simulink model "MCVT_Integration"  (R2020a)
settings: Solver=ode8  StartTime=0.0  StopTime=T_end  FixedStep=dt  RelTol=1e-3  EnableMultiTasking=on
values from neighbouring files (symbols this model uses):
  assumed_power_voltage = 1.25e2    (= 125)    [MCVT_Input_File.m]    (also in MCVT_Run_QuickCheck.m)
  battery_power_toggle = 1    [MCVT_Input_File.m]    (also in MCVT_Run_QuickCheck.m)
  battery_trickle_charge_param = 0.1    [MCVT_Input_File.m]    (also in MCVT_Run_QuickCheck.m)
  battery_trickle_leakage_param = 0.01    [MCVT_Input_File.m]    (also in MCVT_Run_QuickCheck.m)
  damage_case_id = 3    [MCVT_Input_File.m]    (also in MCVT_Run_QuickCheck.m = 1)
  dt = 1/fs    (= 0.001)    [MCVT_Run_QuickCheck.m]    (also in MCVT_Sim_Set.m)
  dt_2 = 1/fs_2    (= 0.001)    [MCVT_Run_QuickCheck.m]    (also in MCVT_Sim_Set.m)
  dt_3 = 1/fs_3    (= 0.001)    [MCVT_Run_QuickCheck.m]    (also in MCVT_Sim_Set.m)
  dt_5 = 1/fs_5    (= 0.005)    [MCVT_Run_QuickCheck.m]    (also in MCVT_Sim_Set.m)
  dt_8 = 1/fs_8    (= 0.05)    [MCVT_Run_QuickCheck.m]    (also in MCVT_Sim_Set.m)
  dt_9 = 1/fs_9    (= 0.001)    [MCVT_Run_QuickCheck.m]    (also in MCVT_Sim_Set.m)
  dust_met_duration = 3    [MCVT_Input_File.m]    (also in MCVT_Run_QuickCheck.m = 2)
  ECLSS_indoor_set_temperature = 298.15    [MCVT_Input_File.m]    (also in MCVT_Run_QuickCheck.m)
  energy_store_max_o = 150    [MCVT_Input_File.m]    (also in MCVT_Run_QuickCheck.m)
  energy_store_o = 120    [MCVT_Input_File.m]    (also in MCVT_Run_QuickCheck.m)
  fs = 1000    [MCVT_Run_QuickCheck.m]    (also in MCVT_Sim_Set.m)
  gain_charge_param = 1    [MCVT_Input_File.m]    (also in MCVT_Run_QuickCheck.m)
  ground_temperature = 373    [MCVT_Habitat_Design_Input_File.m]    (also in MCVT_Run_QuickCheck.m)
  I_max_charge = 800    [MCVT_Input_File.m]    (also in MCVT_Run_QuickCheck.m)
  I_max_discharge = 800    [MCVT_Input_File.m]    (also in MCVT_Run_QuickCheck.m)
  initial_pressure = 1e5    (= 100000)    [MCVT_Input_File.m]    (also in MCVT_Run_QuickCheck.m)
  initial_temperature = 295    [MCVT_Input_File.m]    (also in MCVT_Run_QuickCheck.m)
  interior_leak_rate = 0.00415    [MCVT_Input_File.m]    (also in MCVT_Run_QuickCheck.m)
  launch_dust_accum_start_time = 10    [MCVT_Input_File.m]    (also in MCVT_Run_QuickCheck.m = 2)
  launch_dust_accum_stop_time = 12    [MCVT_Input_File.m]    (also in MCVT_Run_QuickCheck.m = 3)
  meteorite_impact_time = 2    [MCVT_Input_File.m]    (also in MCVT_Run_QuickCheck.m = 1)
  nuclear_power_toggle = 1    [MCVT_Input_File.m]    (also in MCVT_Run_QuickCheck.m)
  print_interval = 30    [MCVT_Input_File.m]
  SOC_lower_thres = 0.5    [MCVT_Input_File.m]    (also in MCVT_Run_QuickCheck.m)
  SOC_upper_thres = 0.9    [MCVT_Input_File.m]    (also in MCVT_Run_QuickCheck.m)
  solar_power_toggle = 1    [MCVT_Input_File.m]    (also in MCVT_Run_QuickCheck.m)
  space_temperature = 4    [MCVT_Habitat_Design_Input_File.m]    (also in MCVT_Run_QuickCheck.m)
  Start_angle = 90    [MCVT_Input_File.m]    (also in MCVT_Run_QuickCheck.m)
undefined symbols (not defined in the file or in its folder): A_flow_restrictor, ACTIVITY_LOOKUP_TABLE, air, Aref, Bmap, Bmap2, Bmap3, comms, DiscIntNeverNeededParam, DmgSPL, dust_parameters, ECLSS_dust_damage_repair_time, ECLSS_heater_capacity, ECLSS_IPS_restrictor, ECLSS_IPS_setpointPressure, ECLSS_paint_damage_repair_time, ECLSS_radiatorLoop_discreteNumber, ECLSS_Vst, emptyActivity, expected_heat_add, FI, habitat, HIEM2SMM, HIEM2STM, HIEMDamageElements, impact_numbering, initial_SPLT_damage_index, input_air, input_Cond, input_CP, input_discretization, input_EV, input_Evap, input_radiatorLoop, K_ECLSS_dust_single, K_ECLSS_paint_single, K_Structure_damage_single, Kref, kW_to_W, meteoroidDustDep, mFRF, ndelays, nominalDustDep, noverlap, nuclear_toggle_gain, output_limits, panel_paint0, Ps0, reference_temperature, RepairTime_ECLSS_factor, Sensor_Location_SMM, SMM_T0, SMM_T1, SMM_T2, SMM_T3, SMM_T4, SMM_T4_0, SMM_T4_1, SMM_T4_2, solar_FDD_K ... (+73)
libraries: neural x350, simulink x39, xpcutilitieslib x7, xpcrtudplib x7, dspstat3 x1
(part 4 of 43)

== system MCVT_Integration/Sys8 - Interior Environment/Habitat Interior Environment Model/Temperature Sensors ==
  note: Sensors_RTD_ECLSS_InputLoc
  note: Sensors_RTD_Zenith
  note: Sensors_RTD_Lef tB ottom
  note: Switch Control
      | 1 - Switch ON
      | 2 - Switch OFF
  note: Switch Control
      | 1 - Switch ON
      | 2 - Switch OFF
  note: Switch Control
      | 1 - Switch ON
      | 2 - Switch OFF
  Temperature (K) : Inport
  Power_Input (kW) : Inport  Port=2
  " Resistance Temperature Detector" : Subsystem
  " Resistance Temperature Detector1" : Subsystem
  " Resistance Temperature Detector2" : Subsystem
  " Switch ON/OFF Control Number" : MATLAB Function  TreatAsAtomicUnit=on
  " Switch ON/OFF Control Number1" : MATLAB Function  TreatAsAtomicUnit=on
  " Switch ON/OFF Control Number2" : MATLAB Function  TreatAsAtomicUnit=on
  Add : Sum  Inputs=+-
  Constant1 : Constant  Value=273.15
  Gain : Gain  Gain=1/3
  Memory3 : Memory  InitialCondition=initial_temperature-273.15  InheritSampleTime=on
  Memory4 : Memory  InitialCondition=initial_temperature-273.15  InheritSampleTime=on
  Memory5 : Memory  InitialCondition=initial_temperature-273.15  InheritSampleTime=on
  Mux : Mux  Inputs=2
  Mux1 : Mux  Inputs=2
  Mux2 : Mux  Inputs=2
  Mux3 : Mux  Inputs=3
  Scope : Scope
  Sensor Input to CDCM : MATLAB Function  TreatAsAtomicUnit=on
  Sensor Placement : MATLAB Function  TreatAsAtomicUnit=on
  Sum : Sum  Inputs=+++  AccumDataTypeStr=double
  Measured_Temperature (K) : Outport
  Power_Consumption (kW) : Outport  Port=2
  ECLSS_Measured_Temperature (K) : Outport  Port=3
  wires:
  " Resistance Temperature Detector2"/1(Physical Interdependency - OUT) -> Mux2/1  "Actual Temperature"
  " Resistance Temperature Detector"/2(Cyber Interdependency - OUT) -> Sensor Input to CDCM/1(ECLSS), ECLSS_Measured_Temperature (K)/1, Mux/2  "Measured Temperature"
  Memory3/1 -> " Resistance Temperature Detector"/1(Physical Interdependency - IN)
  " Switch ON/OFF Control Number1"/1(Switch) -> " Resistance Temperature Detector1"/2(Switch Control)
  Memory5/1 -> " Resistance Temperature Detector2"/1(Physical Interdependency - IN)
  " Resistance Temperature Detector1"/2(Cyber Interdependency - OUT) -> Sensor Input to CDCM/2(Zenith), Mux1/2  "Measured Temperature"
  " Resistance Temperature Detector"/1(Physical Interdependency - OUT) -> Mux/1  "Actual Temperature"
  " Resistance Temperature Detector2"/2(Cyber Interdependency - OUT) -> Sensor Input to CDCM/3(LeftBottom), Mux2/2  "Measured Temperature"
  Sensor Placement/3(LeftBottom) -> Memory5/1
  " Switch ON/OFF Control Number2"/1(Switch) -> " Resistance Temperature Detector2"/2(Switch Control)
  " Resistance Temperature Detector1"/1(Physical Interdependency - OUT) -> Mux1/1  "Actual Temperature"
  Memory4/1 -> " Resistance Temperature Detector1"/1(Physical Interdependency - IN)
  Sensor Placement/2(Zenith) -> Memory4/1
  Sensor Input to CDCM/1(Air_Temp_Sensed) -> Measured_Temperature (K)/1
  " Resistance Temperature Detector"/3(Power_Usage (kW)) -> Mux3/1, Sum/1
  " Resistance Temperature Detector1"/3(Power_Usage (kW)) -> Mux3/2, Sum/2
  " Resistance Temperature Detector2"/3(Power_Usage (kW)) -> Sum/3, Mux3/3
  Sum/1 -> Power_Consumption (kW)/1
  Gain/1 -> " Switch ON/OFF Control Number1"/1(Power), " Resistance Temperature Detector"/3(Power_Supply (kW)), " Switch ON/OFF Control Number"/1(Power), " Resistance Temperature Detector1"/3(Power_Supply (kW)), " Resistance Temperature Detector2"/3(Power_Supply (kW)), " Switch ON/OFF Control Number2"/1(Power)
  Temperature (K)/1 -> Add/1
  Add/1 -> Sensor Placement/1(Air_Temp)
  Constant1/1 -> Add/2
  " Switch ON/OFF Control Number"/1(Switch) -> " Resistance Temperature Detector"/2(Switch Control)
  Power_Input (kW)/1 -> Gain/1
  Sensor Placement/1(ECLSS) -> Scope/1, Memory3/1

== system MCVT_Integration/Sys9 - Exterior Environment/Exterior Environment/Dust ==
  exhaustEvent : Inport
  meteoroidEvent : Inport  Port=2
  nominalDustDep : Inport  Port=3
  meteoroidDustDep : Inport  Port=4
  d : Inport  Port=5
  input_L : Inport  Port=6
  DustDep : MATLAB Function  TreatAsAtomicUnit=on
  Unit Delay1 : UnitDelay  SampleTime=-1  HasFrameUpgradeWarning=on
  Unit Delay2 : UnitDelay  SampleTime=-1  HasFrameUpgradeWarning=on
  tao_total : Outport
  wires:
  d/1 -> DustDep/5(dist)
  input_L/1 -> DustDep/6(input_L)
  DustDep/1(tao_total) -> tao_total/1
  exhaustEvent/1 -> DustDep/1(exhaustEvent)
  nominalDustDep/1 -> DustDep/3(nominalDustDep)
  meteoroidEvent/1 -> DustDep/2(meteoroidEvent)
  meteoroidDustDep/1 -> DustDep/4(meteoroidDustDep)
  DustDep/2(count1) -> Unit Delay1/1
  Unit Delay1/1 -> DustDep/7(countp1)
  DustDep/3(count2) -> Unit Delay2/1
  Unit Delay2/1 -> DustDep/8(countp2)

== system MCVT_Integration/Sys9 - Exterior Environment/Exterior Environment/ExhaustEvent ==
  Add1 : Sum  Inputs=+-
  Step : Step  Time=launch_dust_accum_start_time  SampleTime=dt_9
  Step1 : Step  Time=launch_dust_accum_stop_time  SampleTime=dt_9
  Out1 : Outport
  wires:
  Add1/1 -> Out1/1
  Step/1 -> Add1/1
  Step1/1 -> Add1/2

== system MCVT_Integration/Sys9 - Exterior Environment/Exterior Environment/MeteoroidEvent ==
  Add1 : Sum  Inputs=+-
  Step : Step  Time=meteorite_impact_time  SampleTime=dt_9
  Step1 : Step  Time=meteorite_impact_time+dust_met_duration  SampleTime=dt_9
  meteoroidEvent : Outport
  wires:
  Add1/1 -> meteoroidEvent/1
  Step/1 -> Add1/1
  Step1/1 -> Add1/2

== system MCVT_Integration/Sys 6 - Health Management and Agents/ Agent System/Agent Module/Action Scheduler ==
  CURR_TODO : Inport
  Stateflow Module (re-factored) : Stateflow chart  TreatAsAtomicUnit=on
  Terminator : Terminator
  module1 : MATLAB Function  TreatAsAtomicUnit=on
  currAcvtDone : Outport
  currActID : Outport  Port=2
  currActParams : Outport  Port=3
  currActDone : Outport  Port=4
  actSampleTime : Outport  Port=5
  wires:
  CURR_TODO/1 -> module1/1(program)
  module1/2(todo_pgm) -> Stateflow Module (re-factored)/1(actionData)
  module1/3(nValidActions) -> Stateflow Module (re-factored)/2(numValidActions)
  Stateflow Module (re-factored)/1(actID) -> currActID/1
  Stateflow Module (re-factored)/2(actionParams) -> currActParams/1
  Stateflow Module (re-factored)/3(currAcvtDone) -> currAcvtDone/1
  Stateflow Module (re-factored)/4(currActDone) -> currActDone/1
  Stateflow Module (re-factored)/5(actSampleTime) -> actSampleTime/1
  module1/1(meta_pgm) -> Terminator/1

== system MCVT_Integration/Sys 6 - Health Management and Agents/ Agent System/Agent Module/Agent Memory ==
  currAcvtDone : Inport
  Intervention_Pgm : Inport  Port=2
  Linear Memory Buffer : MATLABSystem  System=LinearMemoryModule  maxMemorySlots=agent_memory_size  numMaxActions=numMaxActions  numMaxActionParams=numMaxActionParams  SimulateUsing=Code generation
      mask Type: LinearMemoryModule
      mask Display:
      | disp('LinearMemoryModule');
      | port_label('input',1,'C2Signal');
      | port_label('input',2,'currAcvtDone');
      | port_label('output',1,'currPgm');
      | port_label('output',2,'filledMemSlots');
      | port_label('output',3,'activityIDs');
      | port_label('output',4,'currAcvtID');
  currPgm : Outport
  filledMemSlots : Outport  Port=2
  activityIDs : Outport  Port=3
  currAcvtID : Outport  Port=4
  wires:
  Intervention_Pgm/1 -> Linear Memory Buffer/1
  Linear Memory Buffer/1 -> currPgm/1
  currAcvtDone/1 -> Linear Memory Buffer/2
  Linear Memory Buffer/2 -> filledMemSlots/1
  Linear Memory Buffer/3 -> activityIDs/1
  Linear Memory Buffer/4 -> currAcvtID/1

== system MCVT_Integration/Sys 6 - Health Management and Agents/ Agent System/Agent Module/Plant Model ==
  actionID : Inport
  actionParams : Inport  Port=2
  actionDone : Inport  Port=3
  actSampleTime : Inport  Port=4
  Agent System Object : MATLABSystem  System=AgentPlant  dt=dt_6  dischargeRate=R_batteryDischarge  mobDamageRate=R_mobDamage  lifeOfAgent=agent_life  SimulateUsing=Code generation
      mask Type: AgentPlant
      mask Display:
      | disp('AgentPlant');
      | port_label('input',1,'actionID');
      | port_label('input',2,'actionParams');
      | port_label('input',3,'actionDone');
      | port_label('input',4,'actSampleTime');
      | port_label('output',1,'States');
      | port_label('output',2,'CommsSubSys_Combined');
      | port_label('output',3,'doneFLAGS');
  States : Outport
  Comms_SubSys_Combined : Outport  Port=2
  action_done_flags : Outport  Port=3
  wires:
  actionID/1 -> Agent System Object/1  "actionID"
  actionDone/1 -> Agent System Object/3  "actionDone"
  actionParams/1 -> Agent System Object/2  "actionParams"
  actSampleTime/1 -> Agent System Object/4
  Agent System Object/1 -> States/1
  Agent System Object/2 -> Comms_SubSys_Combined/1
  Agent System Object/3 -> action_done_flags/1

== system MCVT_Integration/Sys 6 - Health Management and Agents/Decision Maker/Simulink Communication Modules/Agent//MCVT API from HMS ==
  MATLAB Function : MATLAB Function  TreatAsAtomicUnit=on
  Terminator5 : Terminator
  UDP_Receive_C2 : Subsystem
  HMS_Suggested_Activity : Outport
  wires:
  UDP_Receive_C2/2(Receive_output) -> MATLAB Function/1(u)
  UDP_Receive_C2/1(Receive_file) -> Terminator5/1
  UDP_Receive_C2/3(Receive_length) -> MATLAB Function/2(Receive_length)
  MATLAB Function/1(y) -> HMS_Suggested_Activity/1

== system MCVT_Integration/Sys 6 - Health Management and Agents/Decision Maker/Simulink Communication Modules/Agent//MCVT API to HMS ==
  note: Socket Information
  Agent_States : Inport
  Clock1 : Clock
  Constant1 : Constant  Value=comms.send.Agent.DBTableID
  Constant3 : Constant  Value=comms.send.Agent.PacketInfo
  Gain : Gain  Gain=fs
  Terminator : Terminator
  UDP_Send_C2 : Subsystem
  Vector Concatenate : Concatenate  NumInputs=3
  wires:
  Clock1/1 -> Gain/1
  Constant1/1 -> Vector Concatenate/1
  UDP_Send_C2/1(Send_file) -> Terminator/1
  Vector Concatenate/1 -> UDP_Send_C2/1(Input_Send)
  Gain/1 -> Vector Concatenate/2
  Constant3/1 -> UDP_Send_C2/2(Packet_Info)
  Agent_States/1 -> Vector Concatenate/3

== system MCVT_Integration/Sys 6 - Health Management and Agents/Decision Maker/Simulink Communication Modules/ECLSS_Dust//MCVT API to HMS ==
  note: Socket Definition
  note: FDD Information "Summarizer"
  ECLSS_Dust_xe : Inport
  Bus Creator : BusCreator  Inputs=3
  Bus Selector : BusSelector  OutputSignals=FDD_ECLSS_Dust_xe_avg
  Clock1 : Clock
  Constant : Constant  Value=comms.send.ECLSS.FDD_Dust.fsRatio
  Constant3 : Constant  Value=comms.send.ECLSS.FDD_Dust.PacketInfo
  Gain : Gain  Gain=fs
  Terminator : Terminator
  UDP_Send : Subsystem
  Vector Concatenate : Concatenate  NumInputs=3
  module2 : MATLAB Function  TreatAsAtomicUnit=on
  table_id : Constant  Value=comms.send.ECLSS.FDD_Dust.DBTableID
  wires:
  Constant/1 -> module2/2(buffSize)
  Gain/1 -> Vector Concatenate/2
  table_id/1 -> Vector Concatenate/1
  Vector Concatenate/1 -> UDP_Send/1(Input_Send)
  UDP_Send/1(Send_file) -> Terminator/1
  Clock1/1 -> Gain/1
  Constant3/1 -> UDP_Send/2(Packet_Info)
  ECLSS_Dust_xe/1 -> Bus Creator/1, module2/1(u_t)  "FDD_ECLSS_Dust_xe"
  module2/2(u_std) -> Bus Creator/3  "FDD_ECLSS_Dust_xe_std"
  module2/1(u_av) -> Bus Creator/2  "FDD_ECLSS_Dust_xe_avg"
  Bus Creator/1 -> [FDD_ECLSS_Dust_Summary]
  [FDD_ECLSS_Dust_Summary] -> Bus Selector/1
  Bus Selector/1 -> Vector Concatenate/3  "<FDD_ECLSS_Dust_xe_avg>"

== system MCVT_Integration/Sys 6 - Health Management and Agents/Decision Maker/Simulink Communication Modules/ECLSS_Paint//MCVT API to HMS ==
  note: Socket Information
  note: FDD Information "Summarizer"
  ECLSS_Paint_xe : Inport
  Bus Creator : BusCreator  Inputs=3
  Bus Selector : BusSelector  OutputSignals=FDD_ECLSS_Paint_xe_avg
  Clock1 : Clock
  Constant : Constant  Value=comms.send.ECLSS.FDD_Paint.fsRatio
  Constant3 : Constant  Value=comms.send.ECLSS.FDD_Paint.PacketInfo
  Gain : Gain  Gain=fs
  Terminator : Terminator
  UDP_Send : Subsystem
  Vector Concatenate : Concatenate  NumInputs=3
  module2 : MATLAB Function  TreatAsAtomicUnit=on
  table_id : Constant  Value=comms.send.ECLSS.FDD_Paint.DBTableID
  wires:
  Constant/1 -> module2/2(buffSize)
  ECLSS_Paint_xe/1 -> Bus Creator/1, module2/1(u_t)  "FDD_ECLSS_Paint_xe"
  Vector Concatenate/1 -> UDP_Send/1(Input_Send)
  Clock1/1 -> Gain/1
  Gain/1 -> Vector Concatenate/2
  UDP_Send/1(Send_file) -> Terminator/1
  Constant3/1 -> UDP_Send/2(Packet_Info)
  table_id/1 -> Vector Concatenate/1
  module2/1(u_av) -> Bus Creator/2  "FDD_ECLSS_Paint_xe_avg"
  module2/2(u_std) -> Bus Creator/3  "FDD_ECLSS_Paint_xe_std"
  Bus Creator/1 -> [FDD_ECLSS_Paint_Summary]
  [FDD_ECLSS_Paint_Summary] -> Bus Selector/1
  Bus Selector/1 -> Vector Concatenate/3  "<FDD_ECLSS_Paint_xe_avg>"

== system MCVT_Integration/Sys 6 - Health Management and Agents/Decision Maker/Simulink Communication Modules/NPG_Dust//MCVT API to HMS ==
  note: Socket Definition
  note: FDD Information "Summarizer"
  FDD_NPG_Dust : Inport
  Buffer Average : MATLAB Function  TreatAsAtomicUnit=on
  Bus Creator : BusCreator  Inputs=3
  Bus Selector : BusSelector  OutputSignals=FDD_NPG_Dust_avg
  Clock1 : Clock
  Constant : Constant  Value=comms.send.NPG.FDD_Dust.fsRatio
  Constant1 : Constant  Value=comms.send.NPG.FDD_Dust.DBTableID
  Constant3 : Constant  Value=comms.send.NPG.FDD_Dust.PacketInfo
  Gain : Gain  Gain=fs
  Gain1 : Gain  Gain=1/sub3_nuc_FDD_gain
  Terminator1 : Terminator
  UDP_Send : Subsystem
  Vector Concatenate : Concatenate  NumInputs=3
  wires:
  Constant/1 -> Buffer Average/2(buffSize)
  Constant1/1 -> Vector Concatenate/1
  Clock1/1 -> Gain/1
  Gain/1 -> Vector Concatenate/2
  UDP_Send/1(Send_file) -> Terminator1/1
  Constant3/1 -> UDP_Send/2(Packet_Info)
  Vector Concatenate/1 -> UDP_Send/1(Input_Send)
  Buffer Average/1(u_av) -> Bus Creator/2  "FDD_NPG_Dust_avg"
  Buffer Average/2(u_std) -> Bus Creator/3  "FDD_NPG_Dust_std"
  Bus Creator/1 -> [FDD_PG_Dust_Summary]
  [FDD_PG_Dust_Summary] -> Bus Selector/1
  Bus Selector/1 -> Vector Concatenate/3  "<FDD_NPG_Dust_avg>"
  Gain1/1 -> Bus Creator/1, Buffer Average/1(u_t)  "FDD_NPG_Dust"
  FDD_NPG_Dust/1 -> Gain1/1

== system MCVT_Integration/Sys 6 - Health Management and Agents/Decision Maker/Simulink Communication Modules/Solar_PV//MCVT API to HMS ==
  note: Socket Definition
  note: FDD Information "Summarizer"
  FDD_SPG_Dust_xe : Inport
  Buffer Average : MATLAB Function  TreatAsAtomicUnit=on
  Bus Creator : BusCreator  Inputs=3
  Bus Selector : BusSelector  OutputSignals=FDD_SPG_Dust_xe_avg
  Bus Selector1 : BusSelector  OutputSignals=FDD_SPG_Dust_xe,FDD_SPG_Dust_xe_avg,FDD_SPG_Dust_xe_std
  Clock1 : Clock
  Constant : Constant  Value=comms.send.SPG.FDD_Dust.fsRatio
  Constant1 : Constant  Value=comms.send.SPG.FDD_Dust.DBTableID
  Constant3 : Constant  Value=comms.send.SPG.FDD_Dust.PacketInfo
  Gain : Gain  Gain=fs
  Scope : Scope  NumInputPorts=3
  Terminator1 : Terminator
  UDP_Send : Subsystem
  Vector Concatenate : Concatenate  NumInputs=3
  wires:
  FDD_SPG_Dust_xe/1 -> Bus Creator/1, Buffer Average/1(u_t)  "FDD_SPG_Dust_xe"
  Constant/1 -> Buffer Average/2(buffSize)
  Constant1/1 -> Vector Concatenate/1
  Clock1/1 -> Gain/1
  Gain/1 -> Vector Concatenate/2
  UDP_Send/1(Send_file) -> Terminator1/1
  Constant3/1 -> UDP_Send/2(Packet_Info)
  Vector Concatenate/1 -> UDP_Send/1(Input_Send)
  Buffer Average/1(u_av) -> Bus Creator/2  "FDD_SPG_Dust_xe_avg"
  Buffer Average/2(u_std) -> Bus Creator/3  "FDD_SPG_Dust_xe_std"
  Bus Creator/1 -> [FDD_SPG_Dust_Summary]
  [FDD_SPG_Dust_Summary] -> Bus Selector/1
  Bus Selector/1 -> Vector Concatenate/3  "<FDD_SPG_Dust_xe_avg>"
  [FDD_SPG_Dust_Summary] -> Bus Selector1/1
  Bus Selector1/1 -> Scope/1  "<FDD_SPG_Dust_xe>"
  Bus Selector1/2 -> Scope/2  "<FDD_SPG_Dust_xe_avg>"
  Bus Selector1/3 -> Scope/3  "<FDD_SPG_Dust_xe_std>"

== system MCVT_Integration/Sys 6 - Health Management and Agents/Decision Maker/Simulink Communication Modules/Structure_Dmg//MCVT API to HMS ==
  note: Socket Definition
  note: FDD Information "Summarizer"
  Structure_FDD_Damage_xe : Inport
  Buffer Average : MATLAB Function  TreatAsAtomicUnit=on
  Bus Creator : BusCreator  Inputs=3
  Bus Selector : BusSelector  OutputSignals=Str_FDD_Dmg_xe,Str_FDD_Dmg_xe_avg,Str_FDD_Dmg_xe_std
  Bus Selector1 : BusSelector  OutputSignals=Str_FDD_Dmg_xe_avg
  Clock1 : Clock
  Constant : Constant  Value=comms.send.Str.FDD_Dmg.fsRatio
  Constant1 : Constant  Value=comms.send.Str.FDD_Dmg.DBTableID
  Constant3 : Constant  Value=comms.send.Str.FDD_Dmg.PacketInfo
  Gain : Gain  Gain=fs
  Structure Scope : Scope  NumInputPorts=3
  Terminator1 : Terminator
  UDP_Send : Subsystem
  Vector Concatenate : Concatenate  NumInputs=3
  wires:
  Structure_FDD_Damage_xe/1 -> Bus Creator/1, Buffer Average/1(u_t)  "Str_FDD_Dmg_xe"
  Constant/1 -> Buffer Average/2(buffSize)
  Buffer Average/2(u_std) -> Bus Creator/3  "Str_FDD_Dmg_xe_std"
  Constant1/1 -> Vector Concatenate/1
  Clock1/1 -> Gain/1
  Gain/1 -> Vector Concatenate/2
  UDP_Send/1(Send_file) -> Terminator1/1
  Constant3/1 -> UDP_Send/2(Packet_Info)
  Vector Concatenate/1 -> UDP_Send/1(Input_Send)
  Buffer Average/1(u_av) -> Bus Creator/2  "Str_FDD_Dmg_xe_avg"
  Bus Creator/1 -> [FDD_Str_Dmg_Summary]
  [FDD_Str_Dmg_Summary] -> Bus Selector/1
  Bus Selector/1 -> Structure Scope/1  "<Str_FDD_Dmg_xe>"
  Bus Selector/2 -> Structure Scope/2  "<Str_FDD_Dmg_xe_avg>"
  Bus Selector/3 -> Structure Scope/3  "<Str_FDD_Dmg_xe_std>"
  [FDD_Str_Dmg_Summary] -> Bus Selector1/1
  Bus Selector1/1 -> Vector Concatenate/3  "<Str_FDD_Dmg_xe_avg>"

== system MCVT_Integration/Sys2 - Structural System/Sys2_Structural_System/Fault Detection and Diagnosis (FDD) 2.3/Accelerometer ==
  Actural Acceleration : Inport
  Accelerometer Power Supply : Inport  Port=2
  Acceleration Delay : Subsystem
  Accelerometer : Subsystem
  Mux : Mux  Inputs=2
  Selector16 : Selector  InputPortWidth=9  IndexOptions=Index vector (dialog)  Indices=[6]  OutputSizes=1
  Selector17 : Selector  InputPortWidth=9  IndexOptions=Index vector (dialog)  Indices=[6]  OutputSizes=1
  Out2 : Outport
  wires:
  Acceleration Delay/1(Delayed Acceleration) -> Out2/1
  Selector17/1 -> Mux/2  "Measured Acceleration"
  Selector16/1 -> Mux/1  "Actural Acceleration"
  Actural Acceleration/1 -> Selector16/1, Accelerometer/1(Physical Interdependency - IN)
  Accelerometer/1(Cyber Interdependency - OUT) -> Acceleration Delay/1(Measured Acceleration), Selector17/1  "Measured Acceleration"
  Accelerometer Power Supply/1 -> Accelerometer/2(SensorPowerSupply)

== system MCVT_Integration/Sys2 - Structural System/Sys2_Structural_System/Fault Detection and Diagnosis (FDD) 2.3/Data Anomaly Detection ==
  "Measured Acceleration " : Inport
  "Trigger " : TriggerPort  VariantControl=Variant
  Data Anomaly Detection : MATLAB Function  TreatAsAtomicUnit=on
  indicator : Outport
  wires:
  "Measured Acceleration "/1 -> Data Anomaly Detection/1(Acc)
  Data Anomaly Detection/1(indicator) -> indicator/1

== system MCVT_Integration/Sys2 - Structural System/Sys2_Structural_System/Fault Detection and Diagnosis (FDD) 2.3/FRF from Accelerometer 1 ==
  Excitation (White Noise) : Inport
  Acceleration Response : Inport  Port=2
  "Trigger " : TriggerPort  VariantControl=Variant
  Constant : Constant  Value=w
  Constant1 : Constant  Value=mFRF
  Constant2 : Constant  Value=noverlap
  FRF Calculation : MATLAB Function  TreatAsAtomicUnit=on
  Out1 : Outport
  wires:
  Constant2/1 -> FRF Calculation/4(noverlap)
  Constant1/1 -> FRF Calculation/3(m)
  Constant/1 -> FRF Calculation/5(w)
  Acceleration Response/1 -> FRF Calculation/2(acc)
  Excitation (White Noise)/1 -> FRF Calculation/1(exc)
  FRF Calculation/1(frf) -> Out1/1

== system MCVT_Integration/Sys2 - Structural System/Sys2_Structural_System/Fault Detection and Diagnosis (FDD) 2.3/FRF from Accelerometer 2 ==
  Excitation (White Noise) : Inport
  Acceleration Response : Inport  Port=2
  "Trigger " : TriggerPort  VariantControl=Variant
  Constant : Constant  Value=w
  Constant1 : Constant  Value=mFRF
  Constant2 : Constant  Value=noverlap
  FRF Calculation : MATLAB Function  TreatAsAtomicUnit=on
  Out1 : Outport
  wires:
  Excitation (White Noise)/1 -> FRF Calculation/1(exc)
  Acceleration Response/1 -> FRF Calculation/2(acc)
  Constant/1 -> FRF Calculation/5(w)
  Constant1/1 -> FRF Calculation/3(m)
  Constant2/1 -> FRF Calculation/4(noverlap)
  FRF Calculation/1(frf) -> Out1/1

== system MCVT_Integration/Sys2 - Structural System/Sys2_Structural_System/Fault Detection and Diagnosis (FDD) 2.3/FRF from Accelerometer 3 ==
  Excitation (White Noise) : Inport
  Acceleration Response : Inport  Port=2
  "Trigger " : TriggerPort  VariantControl=Variant
  Constant : Constant  Value=w
  Constant1 : Constant  Value=mFRF
  Constant2 : Constant  Value=noverlap
  FRF Calculation : MATLAB Function  TreatAsAtomicUnit=on
  Out1 : Outport
  wires:
  Constant2/1 -> FRF Calculation/4(noverlap)
  Constant1/1 -> FRF Calculation/3(m)
  Constant/1 -> FRF Calculation/5(w)
  Acceleration Response/1 -> FRF Calculation/2(acc)
  Excitation (White Noise)/1 -> FRF Calculation/1(exc)
  FRF Calculation/1(frf) -> Out1/1

== system MCVT_Integration/Sys2 - Structural System/Sys2_Structural_System/Fault Detection and Diagnosis (FDD) 2.3/Pattern Recognition Neural Network ==
  FRF 1 : Inport
  FRF 2 : Inport  Port=2
  FRF 3 : Inport  Port=3
  "Trigger " : TriggerPort  VariantControl=Variant
  Matrix Concatenate : Concatenate  NumInputs=3  Mode=Multidimensional array  ConcatenateDimension=2
  Pattern Recognition Neural Network with 10% Noise : Subsystem
      mask help: Pattern Recognition Neural Network
      mask Display: e = 0.06;c1 = [120 150 230]/255;c2 = ([120 150 230]+0)/383;c3 = ([120 150 230]+128)/383;patch([0 1 1 0],[0 0 1 1],c1);patch([0 1 1 1-e 1-e 0+e],[0 0 1 1-e 0+e 0+e],c2);patch([0 0 1 1-e 0+e 0+e],[0 1 1 1-e 1-e 0+e],c3);plot([0 1 1 0 0],[0 0 1 1 0]);fprintf('NNET');port_label('input',1,' Input');port_label('output',1,'Output ');
  Damage Probabilities : Outport
  wires:
  Matrix Concatenate/1 -> Pattern Recognition Neural Network with 10% Noise/1(Input)
  FRF 1/1 -> Matrix Concatenate/1
  FRF 2/1 -> Matrix Concatenate/2
  FRF 3/1 -> Matrix Concatenate/3
  Pattern Recognition Neural Network with 10% Noise/1(Output) -> Damage Probabilities/1

== system MCVT_Integration/Sys2 - Structural System/Sys2_Structural_System/Fault Detection and Diagnosis (FDD) with Binary Classifer/Accelerometer ==
  Actural Acceleration : Inport
  Accelerometer Power Supply : Inport  Port=2
  Acceleration Delay : Subsystem
  Accelerometer : Subsystem
  Mux : Mux  Inputs=2
  Scope : Scope
  Selector16 : Selector  InputPortWidth=9  IndexOptions=Index vector (dialog)  Indices=[6]  OutputSizes=1
  Selector17 : Selector  InputPortWidth=9  IndexOptions=Index vector (dialog)  Indices=[6]  OutputSizes=1
  To Workspace : ToWorkspace  VariableName=Measured_Acc  SampleTime=-1
  Acceleration_delay : Outport
  Acceleration_origin : Outport  Port=2
  wires:
  Acceleration Delay/1(Delayed Acceleration) -> Acceleration_delay/1
  Selector17/1 -> Mux/2  "Measured Acceleration"
  Selector16/1 -> Mux/1  "Actural Acceleration"
  Actural Acceleration/1 -> Selector16/1, Accelerometer/1(Physical Interdependency - IN)
  Mux/1 -> Scope/1
  Accelerometer/1(Cyber Interdependency - OUT) -> Acceleration_origin/1, Acceleration Delay/1(Measured Acceleration), Selector17/1, To Workspace/1  "Measured Acceleration"
  Accelerometer Power Supply/1 -> Accelerometer/2(SensorPowerSupply)

== system MCVT_Integration/Sys2 - Structural System/Sys2_Structural_System/Fault Detection and Diagnosis (FDD) with Binary Classifer/Data Anomaly Detection ==
  "Measured Acceleration " : Inport
  "Trigger " : TriggerPort  VariantControl=Variant
  Data Anomaly Detection : MATLAB Function  TreatAsAtomicUnit=on
  To Workspace : ToWorkspace  Commented=on  VariableName=max_value  SampleTime=-1
  To Workspace1 : ToWorkspace  Commented=on  VariableName=num_non_zeros  SampleTime=-1
  To Workspace2 : ToWorkspace  Commented=on  VariableName=Data_Anomaly_Indicator  SampleTime=-1
  To Workspace3 : ToWorkspace  Commented=on  VariableName=y  SampleTime=-1
  indicator : Outport
  wires:
  "Measured Acceleration "/1 -> Data Anomaly Detection/1(Acc)
  Data Anomaly Detection/1(indicator) -> To Workspace2/1, indicator/1
  Data Anomaly Detection/2(max_value) -> To Workspace/1
  Data Anomaly Detection/3(num_nan) -> To Workspace1/1
  Data Anomaly Detection/4(y) -> To Workspace3/1

== system MCVT_Integration/Sys2 - Structural System/Sys2_Structural_System/Fault Detection and Diagnosis (FDD) with Binary Classifer/FRF from Accelerometer 1 ==
  Excitation (White Noise) : Inport
  Acceleration Response : Inport  Port=2
  "Trigger " : TriggerPort  VariantControl=Variant
  Constant : Constant  Value=w
  Constant1 : Constant  Value=mFRF
  Constant2 : Constant  Value=noverlap
  FRF Calculation : MATLAB Function  TreatAsAtomicUnit=on
  To Workspace1 : ToWorkspace  VariableName=FRF_Input_Exc_1  SampleTime=-1
  To Workspace2 : ToWorkspace  VariableName=FRF_Input_Acc_1  SampleTime=-1
  To Workspace3 : ToWorkspace  VariableName=FRF_1  SampleTime=-1
  Out1 : Outport
  wires:
  Constant2/1 -> FRF Calculation/4(noverlap)
  Constant1/1 -> FRF Calculation/3(m)
  Constant/1 -> FRF Calculation/5(w)
  Acceleration Response/1 -> FRF Calculation/2(acc), To Workspace2/1
  Excitation (White Noise)/1 -> FRF Calculation/1(exc), To Workspace1/1
  FRF Calculation/1(frf) -> To Workspace3/1, Out1/1

== system MCVT_Integration/Sys2 - Structural System/Sys2_Structural_System/Fault Detection and Diagnosis (FDD) with Binary Classifer/FRF from Accelerometer 2 ==
  Excitation (White Noise) : Inport
  Acceleration Response : Inport  Port=2
  "Trigger " : TriggerPort  VariantControl=Variant
  Constant : Constant  Value=w
  Constant1 : Constant  Value=mFRF
  Constant2 : Constant  Value=noverlap
  FRF Calculation : MATLAB Function  TreatAsAtomicUnit=on
  To Workspace1 : ToWorkspace  VariableName=FRF_Input_Exc_2  SampleTime=-1
  To Workspace2 : ToWorkspace  VariableName=FRF_Input_Acc_2  SampleTime=-1
  To Workspace3 : ToWorkspace  VariableName=FRF_2  SampleTime=-1
  Out1 : Outport
  wires:
  Excitation (White Noise)/1 -> To Workspace1/1, FRF Calculation/1(exc)
  Acceleration Response/1 -> To Workspace2/1, FRF Calculation/2(acc)
  Constant/1 -> FRF Calculation/5(w)
  Constant1/1 -> FRF Calculation/3(m)
  Constant2/1 -> FRF Calculation/4(noverlap)
  FRF Calculation/1(frf) -> To Workspace3/1, Out1/1

== system MCVT_Integration/Sys2 - Structural System/Sys2_Structural_System/Fault Detection and Diagnosis (FDD) with Binary Classifer/FRF from Accelerometer 3 ==
  Excitation (White Noise) : Inport
  Acceleration Response : Inport  Port=2
  "Trigger " : TriggerPort  VariantControl=Variant
  Constant : Constant  Value=w
  Constant1 : Constant  Value=mFRF
  Constant2 : Constant  Value=noverlap
  FRF Calculation : MATLAB Function  TreatAsAtomicUnit=on
  To Workspace1 : ToWorkspace  VariableName=FRF_Input_Exc_3  SampleTime=-1
  To Workspace2 : ToWorkspace  VariableName=FRF_Input_Acc_3  SampleTime=-1
  To Workspace3 : ToWorkspace  VariableName=FRF_3  SampleTime=-1
  Out1 : Outport
  wires:
  Constant2/1 -> FRF Calculation/4(noverlap)
  Constant1/1 -> FRF Calculation/3(m)
  Constant/1 -> FRF Calculation/5(w)
  Acceleration Response/1 -> FRF Calculation/2(acc), To Workspace2/1
  Excitation (White Noise)/1 -> FRF Calculation/1(exc), To Workspace1/1
  FRF Calculation/1(frf) -> To Workspace3/1, Out1/1

== system MCVT_Integration/Sys2 - Structural System/Sys2_Structural_System/Fault Detection and Diagnosis (FDD) with Binary Classifer/Pattern Recognition Neural Network ==
  FRF 1 : Inport
  FRF 2 : Inport  Port=2
  FRF 3 : Inport  Port=3
  "Trigger " : TriggerPort  VariantControl=Variant
  Matrix Concatenate : Concatenate  NumInputs=3  Mode=Multidimensional array  ConcatenateDimension=2
  Segment 1 : Subsystem
      mask help: Pattern Recognition Neural Network
      mask Display: e = 0.06;c1 = [120 150 230]/255;c2 = ([120 150 230]+0)/383;c3 = ([120 150 230]+128)/383;patch([0 1 1 0],[0 0 1 1],c1);patch([0 1 1 1-e 1-e 0+e],[0 0 1 1-e 0+e 0+e],c2);patch([0 0 1 1-e 0+e 0+e],[0 1 1 1-e 1-e 0+e],c3);plot([0 1 1 0 0],[0 0 1 1 0]);fprintf('NNET');port_label('input',1,' Input');port_label('output',1,'Output ');
  Segment 2 (2) : Subsystem
      mask help: Pattern Recognition Neural Network
      mask Display: e = 0.06;c1 = [120 150 230]/255;c2 = ([120 150 230]+0)/383;c3 = ([120 150 230]+128)/383;patch([0 1 1 0],[0 0 1 1],c1);patch([0 1 1 1-e 1-e 0+e],[0 0 1 1-e 0+e 0+e],c2);patch([0 0 1 1-e 0+e 0+e],[0 1 1 1-e 1-e 0+e],c3);plot([0 1 1 0 0],[0 0 1 1 0]);fprintf('NNET');port_label('input',1,' Input');port_label('output',1,'Output ');
  Segment 3 : Subsystem
      mask help: Pattern Recognition Neural Network
      mask Display: e = 0.06;c1 = [120 150 230]/255;c2 = ([120 150 230]+0)/383;c3 = ([120 150 230]+128)/383;patch([0 1 1 0],[0 0 1 1],c1);patch([0 1 1 1-e 1-e 0+e],[0 0 1 1-e 0+e 0+e],c2);patch([0 0 1 1-e 0+e 0+e],[0 1 1 1-e 1-e 0+e],c3);plot([0 1 1 0 0],[0 0 1 1 0]);fprintf('NNET');port_label('input',1,' Input');port_label('output',1,'Output ');
  Segment 4 : Subsystem
      mask help: Pattern Recognition Neural Network
      mask Display: e = 0.06;c1 = [120 150 230]/255;c2 = ([120 150 230]+0)/383;c3 = ([120 150 230]+128)/383;patch([0 1 1 0],[0 0 1 1],c1);patch([0 1 1 1-e 1-e 0+e],[0 0 1 1-e 0+e 0+e],c2);patch([0 0 1 1-e 0+e 0+e],[0 1 1 1-e 1-e 0+e],c3);plot([0 1 1 0 0],[0 0 1 1 0]);fprintf('NNET');port_label('input',1,' Input');port_label('output',1,'Output ');
  Segment 5 : Subsystem
      mask help: Pattern Recognition Neural Network
      mask Display: e = 0.06;c1 = [120 150 230]/255;c2 = ([120 150 230]+0)/383;c3 = ([120 150 230]+128)/383;patch([0 1 1 0],[0 0 1 1],c1);patch([0 1 1 1-e 1-e 0+e],[0 0 1 1-e 0+e 0+e],c2);patch([0 0 1 1-e 0+e 0+e],[0 1 1 1-e 1-e 0+e],c3);plot([0 1 1 0 0],[0 0 1 1 0]);fprintf('NNET');port_label('input',1,' Input');port_label('output',1,'Output ');
  To Workspace1 : ToWorkspace  VariableName=Damage_Probabilities_Segment_2_Noise_10  SampleTime=-1
  To Workspace2 : ToWorkspace  VariableName=Damage_Probabilities_Segment_3_Noise_10  SampleTime=-1
  To Workspace3 : ToWorkspace  VariableName=FRF  SampleTime=-1
  To Workspace4 : ToWorkspace  VariableName=Damage_Probabilities_Segment_4_Noise_10  SampleTime=-1
  To Workspace5 : ToWorkspace  VariableName=Damage_Probabilities_Segment_5_Noise_10  SampleTime=-1
  To Workspace7 : ToWorkspace  VariableName=Damage_Probabilities_Segment_1_Noise_10  SampleTime=-1
  Damage Probabilities Segment 1 : Outport
  Damage Probabilities Segment 2 : Outport  Port=2
  Damage Probabilities Segment 3 : Outport  Port=3
  Damage Probabilities Segment 4 : Outport  Port=4
  Damage Probabilities Segment 5 : Outport  Port=5
  wires:
  Matrix Concatenate/1 -> To Workspace3/1, Segment 3/1(Input), Segment 2 (2)/1(Input), Segment 1/1(Input), Segment 5/1(Input), Segment 4/1(Input)
  FRF 1/1 -> Matrix Concatenate/1
  FRF 2/1 -> Matrix Concatenate/2
  FRF 3/1 -> Matrix Concatenate/3
  Segment 1/1(Output) -> Damage Probabilities Segment 1/1, To Workspace7/1
  Segment 2 (2)/1(Output) -> Damage Probabilities Segment 2/1, To Workspace1/1
  Segment 3/1(Output) -> Damage Probabilities Segment 3/1, To Workspace2/1
  Segment 4/1(Output) -> Damage Probabilities Segment 4/1, To Workspace4/1
  Segment 5/1(Output) -> Damage Probabilities Segment 5/1, To Workspace5/1

== system MCVT_Integration/Sys2 - Structural System/Sys2_Structural_System/Fault Detection and Diagnosis (FDD) with Binary Classifer/Subsystem ==
  Acceleration : Inport
  Repair : Inport  Port=2
  Clock : Clock
  Constant : Constant  Value=dt_2
  Delay4 : Delay  DelayLength=500  InitialCondition=0
  Demux : Demux  Outputs=9
  Enabled Subsystem3 : Subsystem  TreatAsAtomicUnit=on
  Information Fusion1 : MATLAB Function  TreatAsAtomicUnit=on
  Matrix Concatenate : Concatenate  NumInputs=9  Mode=Multidimensional array  ConcatenateDimension=2
  Scope : Scope
  Scope1 : Scope
  Tapped Delay{,1..8} (9 alike) : simulink/Discrete/Tapped Delay  vinit=0.0  samptime=-1  NumDelays=501  DelayOrder=Oldest  includeCurrent=off
  Trigger Condition3 : MATLAB Function  TreatAsAtomicUnit=on
  threshold1 : Constant  Value=0.018
  Impact Location : Outport
  wires:
  Demux/8 -> Tapped Delay7/1
  Tapped Delay5/1 -> Matrix Concatenate/6
  Tapped Delay4/1 -> Matrix Concatenate/5
  Tapped Delay3/1 -> Matrix Concatenate/4
  Demux/2 -> Tapped Delay1/1
  Tapped Delay6/1 -> Matrix Concatenate/7
  Tapped Delay8/1 -> Matrix Concatenate/9
  Tapped Delay7/1 -> Matrix Concatenate/8
  Demux/7 -> Tapped Delay6/1
  Demux/5 -> Tapped Delay4/1
  Tapped Delay1/1 -> Matrix Concatenate/2
  Demux/6 -> Tapped Delay5/1
  Demux/9 -> Tapped Delay8/1
  Tapped Delay2/1 -> Matrix Concatenate/3
  Tapped Delay/1 -> Matrix Concatenate/1
  Demux/3 -> Tapped Delay2/1
  Demux/4 -> Tapped Delay3/1
  Demux/1 -> Tapped Delay/1
  Acceleration/1 -> Demux/1
  Matrix Concatenate/1 -> Enabled Subsystem3/1(image), Trigger Condition3/2(u)
  threshold1/1 -> Enabled Subsystem3/2(thres), Trigger Condition3/1(thres)
  Repair/1 -> Trigger Condition3/3(repair)
  Delay4/1 -> Scope/1, Enabled Subsystem3/Enable
  Trigger Condition3/1(y) -> Delay4/1
  Constant/1 -> Information Fusion1/3(dt)
  Clock/1 -> Information Fusion1/2(T)
  Enabled Subsystem3/1(y) -> Information Fusion1/1(u)
  Information Fusion1/1(y) -> Scope1/1, Impact Location/1

== system MCVT_Integration/Sys2 - Structural System/Sys2_Structural_System/Structural Thermal Model 2.2/STM Temperature Sensors ==
  note: Sensors_RTD_RightBottom
  note: Sensors_RTD_Zenith
  note: Sensors_RTD_Lef tB ottom
  note: Switch Control
      | 1 - Switch ON
      | 2 - Switch OFF
  note: Switch Control
      | 1 - Switch ON
      | 2 - Switch OFF
  note: Switch Control
      | 1 - Switch ON
      | 2 - Switch OFF
  Temperature (K) : Inport
  Power_Input (kW) : Inport  Port=2
  " Resistance Temperature Detector" : Subsystem
  " Resistance Temperature Detector1" : Subsystem
  " Resistance Temperature Detector2" : Subsystem
  " Switch ON/OFF Control Number4" : MATLAB Function  TreatAsAtomicUnit=on
  Add : Sum  Inputs=+-
  Constant1 : Constant  Value=273.15
  Cumulative_Power_STM : MATLAB Function  TreatAsAtomicUnit=on
  Gain : Gain  Gain=1/3
  Memory3 : Memory  InitialCondition=20
  Memory4 : Memory  InitialCondition=20
  Memory5 : Memory  InitialCondition=20
  Mux : Mux  Inputs=2
  Mux1 : Mux  Inputs=2
  Mux2 : Mux  Inputs=2
  Mux3 : Mux  Inputs=3
  STMCumulativePower : ToWorkspace  VariableName=STM_CumulativePowerConsumption  SampleTime=-1
  Sensor Input to CDCM : MATLAB Function  TreatAsAtomicUnit=on
  Sensor Placement : MATLAB Function  TreatAsAtomicUnit=on
  Sum : Sum  Inputs=+++  AccumDataTypeStr=double
  Unit Delay : UnitDelay  SampleTime=-1  HasFrameUpgradeWarning=on
  Unit Delay1 : UnitDelay  SampleTime=-1  HasFrameUpgradeWarning=on
  Measured_Temperature (K) : Outport
  Power_Consumption_by_Sensor (kW) : Outport  Port=2
  Total_Power_Consumption_STM (kW) : Outport  Port=3
  wires:
  " Resistance Temperature Detector2"/1(Physical Interdependency - OUT) -> Mux2/1  "Actual Temperature"
  " Resistance Temperature Detector"/2(Cyber Interdependency - OUT) -> Sensor Input to CDCM/1(RightBottom), Mux/2  "Measured Temperature"
  Memory3/1 -> " Resistance Temperature Detector"/1(Physical Interdependency - IN)
  Memory5/1 -> " Resistance Temperature Detector2"/1(Physical Interdependency - IN)
  " Resistance Temperature Detector1"/2(Cyber Interdependency - OUT) -> Mux1/2, Sensor Input to CDCM/2(Zenith)  "Measured Temperature"
  " Resistance Temperature Detector"/1(Physical Interdependency - OUT) -> Mux/1  "Actual Temperature"
  Sensor Placement/1(RightBottom) -> Memory3/1
  " Resistance Temperature Detector2"/2(Cyber Interdependency - OUT) -> Sensor Input to CDCM/3(LeftBottom), Mux2/2  "Measured Temperature"
  Sensor Placement/3(LeftBottom) -> Memory5/1
  " Switch ON/OFF Control Number4"/1(Switch) -> " Resistance Temperature Detector1"/2(Switch Control), " Resistance Temperature Detector"/2(Switch Control), " Resistance Temperature Detector2"/2(Switch Control)
  " Resistance Temperature Detector1"/1(Physical Interdependency - OUT) -> Mux1/1  "Actual Temperature"
  Memory4/1 -> " Resistance Temperature Detector1"/1(Physical Interdependency - IN)
  Sensor Placement/2(Zenith) -> Memory4/1
  Sensor Input to CDCM/1(Wall_Temp_Sensed) -> Measured_Temperature (K)/1
  Power_Input (kW)/1 -> Gain/1
  " Resistance Temperature Detector"/3(Power_Usage (kW)) -> Mux3/1, Sum/1
  " Resistance Temperature Detector1"/3(Power_Usage (kW)) -> Mux3/2, Sum/2
  Sum/1 -> Total_Power_Consumption_STM (kW)/1, Unit Delay/1
  " Resistance Temperature Detector2"/3(Power_Usage (kW)) -> Sum/3, Mux3/3
  Mux3/1 -> Power_Consumption_by_Sensor (kW)/1
  Unit Delay/1 -> Cumulative_Power_STM/1(TP)
  Unit Delay1/1 -> Cumulative_Power_STM/2(PrevCTP)
  Cumulative_Power_STM/1(CTP) -> STMCumulativePower/1, Unit Delay1/1
  Constant1/1 -> Add/2
  Temperature (K)/1 -> Add/1
  Add/1 -> Sensor Placement/1(Wall_Temp)
  Gain/1 -> " Resistance Temperature Detector1"/3(Power_Supply (kW)), " Resistance Temperature Detector"/3(Power_Supply (kW)), " Resistance Temperature Detector2"/3(Power_Supply (kW)), " Switch ON/OFF Control Number4"/1(Power)

== system MCVT_Integration/Sys3 - Power System/Power Generation Subsystem1/Nuclear Power Generation/Disturbances1 ==
  Bus Creator : BusCreator  Inputs=4
  Chart1 : Stateflow chart  TreatAsAtomicUnit=on
  Sub3_Internal_Disturbance : Outport
  wires:
  Bus Creator/1 -> Sub3_Internal_Disturbance/1
  Chart1/1(row) -> Bus Creator/1  "reactivity_disturbance"
  Chart1/2(prim_flow) -> Bus Creator/2  "Primary Loop Mass Flow Disturbance"
  Chart1/3(sec_flow) -> Bus Creator/3  "Secondary Loop Mass Flow Disturbance"
  Chart1/4(sec_flux) -> Bus Creator/4  "secondary loop fluctuation"

== system MCVT_Integration/Sys3 - Power System/Power Generation Subsystem1/Nuclear Power Generation/SP-100 Power Generation ==
  Sub3_In_Internal_Disturbance : Inport
  Sub3_In_Dust_Accumulation (mg/cm^2) : Inport  Port=2
  Sub3_In_Intervention : Inport  Port=3
  "\tPower Generation" : Subsystem
  FDD Iterative Online Residual Analysis1 : Subsystem
  Terminator : Terminator
  Terminator1 : Terminator
  Sub3_Out_Physical_Interdependency : Outport
  Sub3_Out_Cyber_Interdependency : Outport  Port=2
  Heat Rejection Fraction of Nominal : Outport  Port=3
  wires:
  Sub3_In_Internal_Disturbance/1 -> [internal_dist2]
  [phys_output] -> FDD Iterative Online Residual Analysis1/1(Physical Output SP100)
  [HRF] -> Heat Rejection Fraction of Nominal/1
  Sub3_In_Intervention/1 -> [intervention3]
  Sub3_In_Dust_Accumulation (mg/cm^2)/1 -> [dust_accum3]
  [cyber_output] -> Sub3_Out_Cyber_Interdependency/1
  [power_nom] -> FDD Iterative Online Residual Analysis1/2(power nominal (kW))
  "\tPower Generation"/1(Cyber Output SP100) -> Terminator/1  "Sensed Signal Values"
  "\tPower Generation"/4(Measured Temp Values Nominal (K)) -> Terminator1/1
  [intervention3] -> FDD Iterative Online Residual Analysis1/3(Sub3_In_Intervention)
  [internal_dist2] -> "\tPower Generation"/1(Disturbance)
  [dust_accum3] -> "\tPower Generation"/2(Sub3_In_Dust_Accumulation (mg/cm^2))
  "\tPower Generation"/2(Physical Output SP100) -> [phys_output]
  [phys_output] -> Sub3_Out_Physical_Interdependency/1
  "\tPower Generation"/3(Heat Rejection Fraction of Nominal) -> [HRF]
  "\tPower Generation"/5(Power nominal (kW)) -> [power_nom]
  FDD Iterative Online Residual Analysis1/1(Cyber Output) -> [cyber_output]

== system MCVT_Integration/Sys3 - Power System/Power Generation Subsystem1/Power Distribution and Storage System/Power Delivered to each EPCE ==
  total_power_avail (kW) : Inport
  Sub3_In_Power_Consumption (kW) : Inport  Port=2
  Terminator : Terminator
  power_distribution : MATLAB Function  TreatAsAtomicUnit=on
  p_consumed (kW) : Outport
  wires:
  total_power_avail (kW)/1 -> power_distribution/1(total_power_avail)
  Sub3_In_Power_Consumption (kW)/1 -> power_distribution/2("power_demands ")  "power_demands"
  power_distribution/1(power_demands) -> p_consumed (kW)/1
  power_distribution/2(status_data) -> Terminator/1

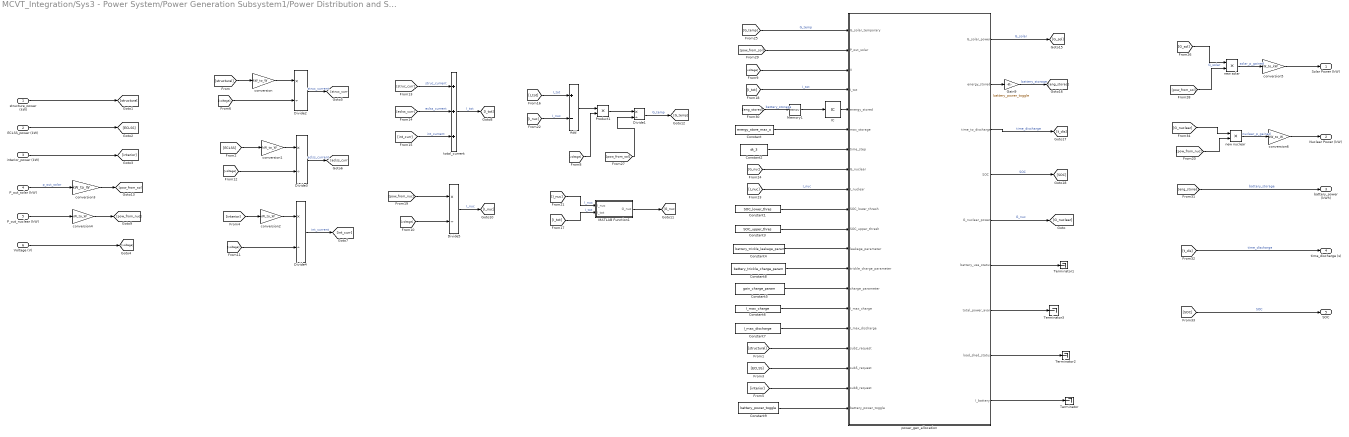
== system MCVT_Integration/Sys3 - Power System/Power Generation Subsystem1/Power Distribution and Storage System/Subsystem ==
  structure_power (kW) : Inport
  ECLSS_power (kW) : Inport  Port=2
  interior_power (kW) : Inport  Port=3
  P_out_solar (kW) : Inport  Port=4
  P_out_nuclear (kW) : Inport  Port=5
  Voltage (V) : Inport  Port=6
  Add : Sum  Inputs=+-
  Constant : Constant  Value=energy_store_max_o
  Constant1 : Constant  Value=SOC_lower_thres
  Constant2 : Constant  Value=dt_3
  Constant3 : Constant  Value=SOC_upper_thres
  Constant4 : Constant  Value=battery_trickle_leakage_param
  Constant5 : Constant  Value=gain_charge_param
  Constant6 : Constant  Value=I_max_charge
  Constant7 : Constant  Value=I_max_discharge
  Constant8 : Constant  Value=battery_trickle_charge_param
  Constant9 : Constant  Value=battery_power_toggle
  Divide{1..5} (5 alike) : Product  Inputs=*/
  Gain9 : Gain  Gain=battery_power_toggle
  IC : InitialCondition  Value=energy_store_o*battery_power_toggle
  MATLAB Function1 : MATLAB Function  TreatAsAtomicUnit=on
  Memory1 : Memory
  Product1 : Product
  Terminator{,1..3} (4 alike) : Terminator
  conversion{,1..4,6} (6 alike) : Gain  Gain=kW_to_W
  conversion5 : Gain  Gain=W_to_kW
  new nuclear : Product
  new solar : Product
  power_gen_allocation : MATLAB Function  TreatAsAtomicUnit=on
  total_current : Sum  Inputs=+++
  Solar Power (kW) : Outport
  Nuclear Power (kW) : Outport  Port=2
  battery_power (kWh) : Outport  Port=3
  time_discharge (s) : Outport  Port=4
  SOC : Outport  Port=5
  wires:
  new nuclear/1 -> conversion6/1  "nuclear_p_gained"
  [G_nuc] -> power_gen_allocation/8(G_nuclear)
  conversion2/1 -> Divide4/1
  conversion/1 -> Divide2/1
  conversion1/1 -> Divide3/1
  [SOC] -> SOC/1  "SOC"
  Memory1/1 -> IC/1
  [t_dis] -> time_discharge (s)/1  "time_discharge"
  power_gen_allocation/5(G_nuclear_power) -> [G_nuclear]  "G_nuc"
  IC/1 -> power_gen_allocation/5("energy_stored ")
  [int_curr] -> total_current/3  "int_current"
  [struc_curr] -> total_current/1  "struc_current"
  [G_sol] -> new solar/1  "G_solar"
  [eclss_curr] -> total_current/2  "eclss_current"
  Product1/1 -> Divide1/1
  [pow_from_nuc] -> Divide5/1
  Constant2/1 -> power_gen_allocation/7(time_step)
  conversion5/1 -> Solar Power (kW)/1
  conversion6/1 -> Nuclear Power (kW)/1
  Constant/1 -> power_gen_allocation/6(max_storage)
  [I_tot] -> Add/1  "I_tot"
  new solar/1 -> conversion5/1  "solar_p_gained"
  [structural] -> conversion/1
  [interior] -> conversion2/1
  Add/1 -> Product1/1
  [ECLSS] -> conversion1/1
  P_out_solar (kW)/1 -> conversion3/1  "p_out_solar"
  [I_nuc] -> MATLAB Function1/1(I_nuc)  "I_nuc"
  power_gen_allocation/2(energy_stored) -> Gain9/1
  [voltage] -> Divide2/2
  [eng_stored] -> battery_power (kWh)/1  "battery_storage"
  Constant7/1 -> power_gen_allocation/16(I_max_discharge)
  Constant6/1 -> power_gen_allocation/15(I_max_charge)
  Constant5/1 -> power_gen_allocation/14(charge_parameter)
  Constant4/1 -> power_gen_allocation/12(leakage_parameter)
  Constant3/1 -> power_gen_allocation/11(SOC_upper_thresh)
  Constant1/1 -> power_gen_allocation/10(SOC_lower_thresh)
  power_gen_allocation/9(I_battery) -> Terminator/1
  Constant8/1 -> power_gen_allocation/13(trickle_charge_parameter)
  Constant9/1 -> power_gen_allocation/20(battery_power_toggle)
  structure_power (kW)/1 -> [structural]
  [structural] -> power_gen_allocation/17(sub2_request)
  ECLSS_power (kW)/1 -> [ECLSS]
  [ECLSS] -> power_gen_allocation/18(sub5_request)
  interior_power (kW)/1 -> [interior]
  [interior] -> power_gen_allocation/19(sub8_request)
  Voltage (V)/1 -> [voltage]
  [voltage] -> Product1/2
  [voltage] -> power_gen_allocation/3(V)
  [voltage] -> Divide5/2
  [voltage] -> Divide4/2
  [voltage] -> Divide3/2
  Divide2/1 -> [struc_curr]  "struc_current"
  Divide3/1 -> [eclss_curr]  "eclss_current"
  Divide4/1 -> [int_curr]  "int_current"
  total_current/1 -> [I_tot]  "I_tot"
  [I_tot] -> MATLAB Function1/2(I_tot)  "I_tot"
  [I_tot] -> power_gen_allocation/4(I_tot)  "I_tot"
  P_out_nuclear (kW)/1 -> conversion4/1
  conversion4/1 -> [pow_from_nuc]
  [pow_from_nuc] -> new nuclear/2
  Divide5/1 -> [I_nuc]  "I_nuc"
  [I_nuc] -> Add/2  "I_nuc"
  [I_nuc] -> power_gen_allocation/9(I_nuclear)  "I_nuc"
  MATLAB Function1/1(G_nuc) -> [G_nuc]
  Divide1/1 -> [G_temp]  "G_temp"
  conversion3/1 -> [pow_from_sol]
  [pow_from_sol] -> Divide1/2
  [pow_from_sol] -> new solar/2
  [pow_from_sol] -> power_gen_allocation/2(P_out_solar)
  power_gen_allocation/1(G_solar_power) -> [G_sol]  "G_solar"
  Gain9/1 -> [eng_stored]  "battery_storage"
  [eng_stored] -> Memory1/1  "battery_storage"
  power_gen_allocation/3(time_to_discharge) -> [t_dis]  "time_discharge"
  power_gen_allocation/4(SOC) -> [SOC]  "SOC"
  [G_nuclear] -> new nuclear/1
  power_gen_allocation/7(total_power_avail) -> Terminator3/1
  power_gen_allocation/6(battery_use_status) -> Terminator1/1
  power_gen_allocation/8(load_shed_status) -> Terminator2/1
  [G_temp] -> power_gen_allocation/1(G_solar_temporary)  "G_temp"
